simulink model "CCMLin2Mgr"  (R2022b) (continued)
== system CCMLin2Mgr/Lin2Mgr_Runnable_sys == (continued)
  SysServCarCfgSts_SysServDUDCSTLSts_read/1 -> Terminator_18/1
  SysServCarCfgSts_SysServDUDCTLSts_read/1 -> Terminator_19/1
  SysServCarCfgSts_SysServDUDCTRSts_read/1 -> Terminator_20/1
  SysServCarCfgSts_SysServDUFLFootSts_read/1 -> Terminator_21/1
  SysServCarCfgSts_SysServDUFLVentSts_read/1 -> Terminator_22/1
  SysServCarCfgSts_SysServDUFRFootSts_read/1 -> Terminator_23/1
  SysServCarCfgSts_SysServDUFRVentSts_read/1 -> Terminator_24/1
  SysServCarCfgSts_SysServDUIncarMotorSts_read/1 -> Terminator_25/1
  SysServCarCfgSts_SysServDULin1HBMFSts_read/1 -> Terminator_26/1
  SysServCarCfgSts_SysServDULin4HFAFSts_read/1 -> Terminator_27/1
  SysServCarCfgSts_SysServDUSFLFootSts_read/1 -> Terminator_28/1
  SysServCarCfgSts_SysServDUSFLVentSts_read/1 -> Terminator_29/1
  SysServCarCfgSts_SysServDUSFRFootSts_read/1 -> Terminator_30/1
  SysServCarCfgSts_SysServDUSFRVentSts_read/1 -> Terminator_31/1
  SysServCarCfgSts_SysServDUSnsrForFCmptmtTSts_read/1 -> Terminator_32/1
  SysServCarCfgSts_SysServDUSnsrForRair_read/1 -> Terminator_33/1
  SysServCarCfgSts_SysServDUSnsrForRCmptmtTSts_read/1 -> Terminator_34/1
  AACAirClngErrSts_AACAirClngErrSts_read/1 -> Terminator_35/1
  AFUGenErr_AFUGenErr_read/1 -> Terminator_36/1
  AFUPartNo10Cmpl_AFUPartNo10Cmpl_read/1 -> Terminator_37/1
  AFUPartNoCmpl_AFUPartNoCmpl_read/1 -> Terminator_38/1
  AFUSerNo_AFUSerNo_read/1 -> Terminator_39/1
  AirClngErrSts_AirClngErrSts_read/1 -> Terminator_40/1
  AirFragFanErr_AirFragFanErr_read/1 -> Terminator_41/1
  AirFragMot1Err_AirFragMot1Err_read/1 -> Terminator_42/1
  AirFragMot2Err_AirFragMot2Err_read/1 -> Terminator_43/1
  AirFragMot3Err_AirFragMot3Err_read/1 -> Terminator_44/1
  AirFragMot4Err_AirFragMot4Err_read/1 -> Terminator_45/1
  AirFragMot5Err_AirFragMot5Err_read/1 -> Terminator_46/1
  AirFragOvrVoltErr_AirFragOvrVoltErr_read/1 -> Terminator_47/1
  AirFragTempErr_AirFragTempErr_read/1 -> Terminator_48/1
  AirFragUndVoltErr_AirFragUndVoltErr_read/1 -> Terminator_49/1
  AIUPartNo10Cmpl_AIUPartNo10Cmpl_read/1 -> Terminator_50/1
  AIUPartNoCmpl_AIUPartNoCmpl_read/1 -> Terminator_51/1
  AIUSerNo_AIUSerNo_read/1 -> Terminator_52/1
  AQSAirQuality_AQSAirQuality_read/1 -> Terminator_53/1
  AQSCOxstatus_AQSCOxstatus_read/1 -> Terminator_54/1
  AQSNH3status_AQSNH3status_read/1 -> Terminator_55/1
  AQSNoxStatus_AQSNoxStatus_read/1 -> Terminator_56/1
  CarCfgForClimaCtrl_CarCfgForAirClng_read/1 -> Terminator_57/1
  CarCfgForClimaCtrl_CarCfgForAirClnrAdv_read/1 -> Terminator_58/1
  CarCfgForClimaCtrl_CarCfgForAirFrag_read/1 -> Terminator_59/1
  CarCfgForClimaCtrl_CarCfgForAirMtrlSnsr_read/1 -> Terminator_60/1
  CarCfgForClimaCtrl_CarCfgForAirQly_read/1 -> Terminator_61/1
  CarCfgForClimaCtrl_CarCfgForAmntOfSeat_read/1 -> Terminator_62/1
  CarCfgForClimaCtrl_CarCfgForAutDemst_read/1 -> Terminator_63/1
  CarCfgForClimaCtrl_CarCfgForAuxWtrPmp_read/1 -> Terminator_64/1
  CarCfgForClimaCtrl_CarCfgForBRAND_read/1 -> Terminator_65/1
  CarCfgForClimaCtrl_CarCfgForBypVlvInHvac_read/1 -> Terminator_66/1
  CarCfgForClimaCtrl_CarCfgForCabinOxgenSupply_read/1 -> Terminator_67/1
  CarCfgForClimaCtrl_CarCfgForClsVlvInHvac_read/1 -> Terminator_68/1
  CarCfgForClimaCtrl_CarCfgForCmprAcTyp_read/1 -> Terminator_69/1
  CarCfgForClimaCtrl_CarCfgForCmptmtClng_read/1 -> Terminator_70/1
  CarCfgForClimaCtrl_CarCfgForElectricLiftingModule_read/1 -> Terminator_71/1
  CarCfgForClimaCtrl_CarCfgForElecVentAdjmt_read/1 -> Terminator_72/1
  CarCfgForClimaCtrl_CarCfgForExtVentnClimaCtrl_read/1 -> Terminator_73/1
  CarCfgForClimaCtrl_CarCfgForFlcTyp_read/1 -> Terminator_74/1
  CarCfgForClimaCtrl_CarCfgForFoldCSD_read/1 -> Terminator_75/1
  CarCfgForClimaCtrl_CarCfgForFuTyp_read/1 -> Terminator_76/1
  CarCfgForClimaCtrl_CarCfgForGlassTyp_read/1 -> Terminator_77/1
  CarCfgForClimaCtrl_CarCfgForHeadRadar_read/1 -> Terminator_78/1
  CarCfgForClimaCtrl_CarCfgForHeatPmp_read/1 -> Terminator_79/1
  CarCfgForClimaCtrl_CarCfgForHeatrDurgDrvg_read/1 -> Terminator_80/1
  CarCfgForClimaCtrl_CarCfgForHeatrTyp_read/1 -> Terminator_81/1
  CarCfgForClimaCtrl_CarCfgForHvacFilTyp_read/1 -> Terminator_82/1
  CarCfgForClimaCtrl_CarCfgForHvacFlrSnsrForT_read/1 -> Terminator_83/1
  CarCfgForClimaCtrl_CarCfgForHvacHeatrSnsrT_read/1 -> Terminator_84/1
  CarCfgForClimaCtrl_CarCfgForHvacRe_read/1 -> Terminator_85/1
  CarCfgForClimaCtrl_CarCfgForHvacTyp_read/1 -> Terminator_86/1
  CarCfgForClimaCtrl_CarCfgForInteriorViewCamera_read/1 -> Terminator_87/1
  CarCfgForClimaCtrl_CarCfgForLifeDetection_read/1 -> Terminator_88/1
  CarCfgForClimaCtrl_CarCfgForLoVoltElecCfg_read/1 -> Terminator_89/1
  CarCfgForClimaCtrl_CarCfgForNavSysandIntnmap_read/1 -> Terminator_90/1
  CarCfgForClimaCtrl_CarCfgForNrOfDoor_read/1 -> Terminator_91/1
  CarCfgForClimaCtrl_CarCfgForPipeInFuTank_read/1 -> Terminator_92/1
  CarCfgForClimaCtrl_CarCfgForPowerSeats_read/1 -> Terminator_93/1
  CarCfgForClimaCtrl_CarCfgForPreClng_read/1 -> Terminator_94/1
  CarCfgForClimaCtrl_CarCfgForPrkgCDSNotAvl_read/1 -> Terminator_95/1
  CarCfgForClimaCtrl_CarCfgForPrkgClima_read/1 -> Terminator_96/1
  CarCfgForClimaCtrl_CarCfgForPrpsnTyp_read/1 -> Terminator_97/1
  CarCfgForClimaCtrl_CarCfgForPTCInFrontHVAC_read/1 -> Terminator_98/1
  CarCfgForClimaCtrl_CarCfgForRemEngStrtPreCdn_read/1 -> Terminator_99/1
  CarCfgForClimaCtrl_CarCfgForRoofHatch_read/1 -> Terminator_100/1
  CarCfgForClimaCtrl_CarCfgForSeatHeatgCal_read/1 -> Terminator_101/1
  CarCfgForClimaCtrl_CarCfgForSeatHeatgForRowFirst_read/1 -> Terminator_102/1
  CarCfgForClimaCtrl_CarCfgForSeatHeatgForRowSec_read/1 -> Terminator_103/1
  CarCfgForClimaCtrl_CarCfgForSeatVentnForRowFirst_read/1 -> Terminator_104/1
  CarCfgForClimaCtrl_CarCfgForSeatVentnForRowSec_read/1 -> Terminator_105/1
  CarCfgForClimaCtrl_CarCfgForSecRowVentnCenter_read/1 -> Terminator_106/1
  CarCfgForClimaCtrl_CarCfgForSecRowVentnSide_read/1 -> Terminator_107/1
  CarCfgForClimaCtrl_CarCfgForSmallFlowCyc_read/1 -> Terminator_108/1
  CarCfgForClimaCtrl_CarCfgForSmkSnsr_read/1 -> Terminator_109/1
  CarCfgForClimaCtrl_CarCfgForSnsrForCmptmtT_read/1 -> Terminator_110/1
  CarCfgForClimaCtrl_CarCfgForSnsrHumPrsnt_read/1 -> Terminator_111/1
  CarCfgForClimaCtrl_CarCfgForStartDevice_read/1 -> Terminator_112/1
  CarCfgForClimaCtrl_CarCfgForSteerWhlHeatg_read/1 -> Terminator_113/1
  CarCfgForClimaCtrl_CarCfgForSteerWhlPosn_read/1 -> Terminator_114/1
  CarCfgForClimaCtrl_CarCfgForSunSnsr_read/1 -> Terminator_115/1
  CarCfgForClimaCtrl_CarCfgForTelmNodTyp_read/1 -> Terminator_116/1
  CarCfgForClimaCtrl_CarCfgForThermMngt_read/1 -> Terminator_117/1
  CarCfgForClimaCtrl_CarCfgForVehTyp_read/1 -> Terminator_118/1
  CarCfgForClimaCtrl_CarCfgForVentLiftMotorExiSts_read/1 -> Terminator_119/1
  CarCfgForClimaCtrl_CarCfgForVgaMotorExistSts_read/1 -> Terminator_120/1
  CarCfgForClimaCtrl_CarCfgForWadingAssistSystem_read/1 -> Terminator_121/1
  CarCfgForClimaCtrl_CarCfgForWindHeatd_read/1 -> Terminator_122/1
  CarCfgForClimaCtrl_CarCfgWindFollowHumanMov_read/1 -> Terminator_123/1
  CDSPartNo10Cmpl_CDSPartNo10Cmpl_read/1 -> Terminator_124/1
  CDSPartNoCmpl_CDSPartNoCmpl_read/1 -> Terminator_125/1
  CDSSerNo_CDSSerNo_read/1 -> Terminator_126/1
  ClimRlyCmd_ClimRlyCmd_read/1 -> Terminator_127/1
  CmpmtInAirPmAQI_CmpmtInAirPmAQI_read/1 -> Terminator_128/1
  CmpmtInAirPmDnsty_CmpmtInAirPmDnsty_read/1 -> Terminator_129/1
  CmptmtOxygenConcQFNgcc_CmptmtOxygenConcQFNgcc_read/1 -> Terminator_130/1
  CmptmtOxygenConcVluNgcc_CmptmtOxygenConcVluNgcc_read/1 -> Terminator_131/1
  HvacCfgForClimaCtrl_HvacCfgForAQSTypes_read/1 -> Terminator_132/1
  HvacCfgForClimaCtrl_HvacCfgForHvacFrontBlowerType_read/1 -> Terminator_133/1
  HvacCfgForClimaCtrl_HvacCfgForHvacLowSideRETXVESts_read/1 -> Terminator_134/1
  HvacCfgForClimaCtrl_HvacCfgForHvacMiscExistSts_read/1 -> Terminator_135/1
  HvacCfgForClimaCtrl_HvacCfgForHvacMotorExistSts_read/1 -> Terminator_136/1
  HvacCfgForClimaCtrl_HvacCfgForHvacReBlowerType_read/1 -> Terminator_137/1
  HvacCfgForClimaCtrl_HvacCfgForHvacReVentPstn_read/1 -> Terminator_138/1
  HvacCfgForClimaCtrl_HvacCfgForHvacSensorExistSts_read/1 -> Terminator_139/1
  HvacCfgForClimaCtrl_HvacCfgForHvacSnsrRCmptmtESts_read/1 -> Terminator_140/1
  HvacCfgForClimaCtrl_HvacCfgForHvacSnsrReHexESts_read/1 -> Terminator_141/1
  HvacFanReqRe_HvacFanReqRe_read/1 -> Terminator_142/1
  HvacFanSts2HvacFanBattU2_HvacFanSts2HvacFanBattU2_read/1 -> Terminator_143/1
  HvacFanSts2HvacFanExt2_HvacFanSts2HvacFanExt2_read/1 -> Terminator_144/1
  HvacFanSts2HvacFanHw2_HvacFanSts2HvacFanHw2_read/1 -> Terminator_145/1
  HvacFanSts2HvacFanMotU2_HvacFanSts2HvacFanMotU2_read/1 -> Terminator_146/1
  HvacFanSts2HvacFanReSts1_HvacFanSts2HvacFanReSts1_read/1 -> Terminator_147/1
  HvacFanSts2HvacFanReSts2_HvacFanSts2HvacFanReSts2_read/1 -> Terminator_148/1
  HvacFanSts2HvacFanSw2_HvacFanSts2HvacFanSw2_read/1 -> Terminator_149/1
  HvacFanSts2HvacFanT2_HvacFanSts2HvacFanT2_read/1 -> Terminator_150/1
  HvacFanVoltReqRe_HvacFanVoltReqRe_read/1 -> Terminator_151/1
  InPm25FanErr_InPm25FanErr_read/1 -> Terminator_152/1
  InPm25SensrIntErr_InPm25SensrIntErr_read/1 -> Terminator_153/1
  InPm25SnrTempErr_InPm25SnrTempErr_read/1 -> Terminator_154/1
  InPm25SnrVoltgRangErr_InPm25SnrVoltgRangErr_read/1 -> Terminator_155/1
  InPm25SnsrElecErr_InPm25SnsrElecErr_read/1 -> Terminator_156/1
  InPm25SnsrSts_InPm25SnsrSts_read/1 -> Terminator_157/1
  IntCo2CircElecErr_IntCo2CircElecErr_read/1 -> Terminator_158/1
  IntCo2HvErr_IntCo2HvErr_read/1 -> Terminator_159/1
  IntCo2LvErr_IntCo2LvErr_read/1 -> Terminator_160/1
  KeepComActvL2_KeepComActvL2_read/1 -> Terminator_161/1
  OxygenConcSnsrElecErr_OxygenConcSnsrElecErr_read/1 -> Terminator_162/1
  OxygenConcSnsrElecErrNgcc_OxygenConcSnsrElecErrNgcc_read/1 -> Terminator_163/1
  OxygenConcSnsrIntErr_OxygenConcSnsrIntErr_read/1 -> Terminator_164/1
  OxygenConcSnsrIntErrNgcc_OxygenConcSnsrIntErrNgcc_read/1 -> Terminator_165/1
  OxygenConcSnsrOvrVoltRangErr_OxygenConcSnsrOvrVoltRangErr_read/1 -> Terminator_166/1
  OxygenConcSnsrStsNgcc_OxygenConcSnsrStsNgcc_read/1 -> Terminator_167/1
  OxygenConcSnsrTempErr_OxygenConcSnsrTempErr_read/1 -> Terminator_168/1
  OxygenConcSnsrTempErrNgcc_OxygenConcSnsrTempErrNgcc_read/1 -> Terminator_169/1
  OxygenConcSnsrUndVoltRangErr_OxygenConcSnsrUndVoltRangErr_read/1 -> Terminator_170/1
  OxygenConcSnsrUndVoltRangErrNgcc_OxygenConcSnsrUndVoltRangErrNgcc_read/1 -> Terminator_171/1
  PMSIPartNo10Hw_PMSIPartNo10Hw_read/1 -> Terminator_172/1
  PMSIPartNoHw_PMSIPartNoHw_read/1 -> Terminator_173/1
  PwrSplyToSnsrForAirQly_PwrSplyToSnsrForAirQly_read/1 -> Terminator_174/1
  RHFAScheTableReq_Val_read/1 -> Terminator_175/1
  SerNoPMSI_SerNoPMSI_read/1 -> Terminator_176/1
  SysServCarCfgSts_SysServCCPBGMNum_read/1 -> Terminator_177/1
  SysServCarCfgSts_SysServCCPLin2AQSSts_read/1 -> Terminator_178/1
  SysServCarCfgSts_SysServDUDCFLMdeSts_read/1 -> Terminator_179/1
  SysServCarCfgSts_SysServDUReBlowerPwmSignSts_read/1 -> Terminator_180/1
  SysServCarCfgSts_SysServDUReBlowerPwmSts_read/1 -> Terminator_181/1
  SysServCarCfgSts_SysServFrntHEXSnsrPosn_read/1 -> Terminator_182/1
  SysServCarCfgSts_SysServLowSideRETXVSts_read/1 -> Terminator_183/1
  SysServCarCfgSts_SysServReHEXSnsrPosn_read/1 -> Terminator_184/1
  SysServCarCfgSts_SysServRHFASts_read/1 -> Terminator_185/1
  SysServCarCfgSts_SysServSnsrForFCmptmtTType_read/1 -> Terminator_186/1
  BswLifeCycle_LifeCycle_read/1 -> Terminator_187/1
  Ground_1/1 -> LinIfServ_GetScheduleInfo/1
  LinIfServ_GetScheduleInfo/1 -> Terminator_188/1
  LinIfServ_GetScheduleInfo/2 -> Terminator_189/1
  Ground_2/1 -> LinSMServ_ScheduleRequest/1
  Ground_3/1 -> LinSMServ_ScheduleRequest/2
  LinSMServ_ScheduleRequest/1 -> Terminator_190/1
  LinService_GetLin2WakeupStatus/1 -> Terminator_191/1
  LinService_GetLin2WakeupStatus/2 -> Terminator_192/1
  Ground_4/1 -> LinService_SendLin3dFrame/1
  Ground_5/1 -> LinService_SendLin3dFrame/2
  LinService_SendLin3dFrame/1 -> Terminator_193/1
  Ground_6/1 -> LinService_SetLin3cFrame/1
  Ground_7/1 -> LinService_SetLin3cFrame/2
  LinService_SetLin3cFrame/1 -> Terminator_194/1
  Ground_8/1 -> LinService_SetLinTable/1
  Ground_9/1 -> LinService_SetLinTable/2
  LinService_SetLinTable/1 -> Terminator_195/1
  MirrorLin2ErrServ_GetAQSCcmLin2Fr01NoResp/1 -> Terminator_196/1
  MirrorLin2ErrServ_GetAQSRxErrSta/1 -> Terminator_197/1
  MirrorLin2ErrServ_GetAfuCcmLin2Fr01NoResp/1 -> Terminator_198/1
  MirrorLin2ErrServ_GetAfuCcmLin2Fr01NoResp/2 -> Terminator_199/1
  MirrorLin2ErrServ_GetAfuRxErrSta/1 -> Terminator_200/1
  MirrorLin2ErrServ_GetAfuRxErrSta/2 -> Terminator_201/1
  MirrorLin2ErrServ_GetAiuCcmLin2Fr01NoResp/1 -> Terminator_202/1
  MirrorLin2ErrServ_GetAiuCcmLin2Fr01NoResp/2 -> Terminator_203/1
  MirrorLin2ErrServ_GetAiuRxErrSta/1 -> Terminator_204/1
  MirrorLin2ErrServ_GetAiuRxErrSta/2 -> Terminator_205/1
  MirrorLin2ErrServ_GetCdsCcmLin2Fr01NoResp/1 -> Terminator_206/1
  MirrorLin2ErrServ_GetCdsCcmLin2Fr01NoResp/2 -> Terminator_207/1
  MirrorLin2ErrServ_GetCdsRxErrSta/1 -> Terminator_208/1
  MirrorLin2ErrServ_GetCdsRxErrSta/2 -> Terminator_209/1
  MirrorLin2ErrServ_GetLinBusTxErrSta/1 -> Terminator_210/1
  MirrorLin2ErrServ_GetLinBusTxErrSta/2 -> Terminator_211/1
  MirrorLin2ErrServ_GetPmsiCcmLin2Fr01NoResp/1 -> Terminator_212/1
  MirrorLin2ErrServ_GetPmsiCcmLin2Fr01NoResp/2 -> Terminator_213/1
  MirrorLin2ErrServ_GetPmsiRxErrSta/1 -> Terminator_214/1
  MirrorLin2ErrServ_GetPmsiRxErrSta/2 -> Terminator_215/1
  MirrorLin2ErrServ_GetRhfaCcmLin2Fr01NoResp/1 -> Terminator_216/1
  MirrorLin2ErrServ_GetRhfaCcmLin2Fr01NoResp/2 -> Terminator_217/1
  MirrorLin2ErrServ_GetRhfaRxErrSta/1 -> Terminator_218/1
  MirrorLin2ErrServ_GetRhfaRxErrSta/2 -> Terminator_219/1
  MirrorLin2ErrServ_GetVbmrCcmLin2Fr01NoResp/1 -> Terminator_220/1
  MirrorLin2ErrServ_GetVbmrCcmLin2Fr01NoResp/2 -> Terminator_221/1
  MirrorLin2ErrServ_GetVbmrRxErrSta/1 -> Terminator_222/1
  MirrorLin2ErrServ_GetVbmrRxErrSta/2 -> Terminator_223/1
  Ground_10/1(Out1) -> AirQlyLin_AirQlyLin_write/1
  Ground_11/1 -> HvacFanSet2HvacFanRxFrq2_HvacFanSet2HvacFanRxFrq2_write/1
  Ground_12/1 -> Lin2ActvReady_Lin2ActvReady_write/1
  Ground_13/1 -> Lin2MgrDtcServ2_AACGenElectrFail_write/1
  Ground_14/1 -> Lin2MgrDtcServ2_IOSMGenElectrFail_write/1
  Ground_15/1 -> Lin2MgrDtcServ2_IOSMInterFail_write/1
  Ground_16/1 -> ReVbmrSts_ReVbmrSts_write/1
  Ground_17/1 -> AirFragErrSts_AirFragErrSts_write/1
  Ground_18/1 -> AirPmQf_AirPmQf_write/1
  Ground_19/1 -> HvacFanSet2HvacFanIMaxMax2_HvacFanSet2HvacFanIMaxMax2_write/1
  Ground_20/1 -> HvacFanSet2HvacFanIMaxMin2_HvacFanSet2HvacFanIMaxMin2_write/1
  Ground_21/1 -> HvacFanSet2HvacFanKneeU2_HvacFanSet2HvacFanKneeU2_write/1
  Ground_22/1 -> HvacFanSet2HvacFanRamp2_HvacFanSet2HvacFanRamp2_write/1
  Ground_23/1 -> HvacFanSet2HvacFanSeln2_HvacFanSet2HvacFanSeln2_write/1
  Ground_24/1 -> HvacFanSet2HvacFanSftyRunThd2_HvacFanSet2HvacFanSftyRunThd2_write/1
  Ground_25/1 -> HvacFanSet2HvacFanSpd2_HvacFanSet2HvacFanSpd2_write/1
  Ground_26/1 -> HvacFanSet2HvacSftyRunSpd2_HvacFanSet2HvacSftyRunSpd2_write/1
  Ground_27/1 -> IntPm25LvlFrmClima_IntPm25LvlFrmClima_write/1
  Ground_28/1 -> IntPm25StsFrmClima_IntPm25StsFrmClima_write/1
  Ground_29/1 -> IntPm25VluFrmClima_IntPm25VluFrmClima_write/1
  Ground_30/1 -> LIN2ScheTableReq_Val_write/1

== system CCMLin2Mgr/Lin2Mgr_SerNoPMSI_sys ==
  SerNoPMSI_SerNoPMSI_read : Inport
  function : TriggerPort  TriggerType=function-call  VariantControl=(inherit)
  Terminator_1 : Terminator
  wires:
  SerNoPMSI_SerNoPMSI_read/1 -> Terminator_1/1

== system CCMLin2Mgr/Lin2ParaService ==
  Lin2ParaService_FreezeVbmrReqPercent_sys : Subsystem
  Lin2ParaService_ReadVbmrPercent_sys : Subsystem
  Lin2ParaService_VbmrReqPercentRetCtrltoECU_sys : Subsystem
  Lin2ParaService_VbmrReqPercentShortTermAdj_sys : Subsystem

== system CCMLin2Mgr/Lin2MgrDtcServ/Lin2MgrDtcServ_AFUGenElectrFail_sys ==
  function : TriggerPort  TriggerType=function-call  IsSimulinkFunction=on  FunctionName=Lin2MgrDtcServ_AFUGenElectrFail  VariantControl=(inherit)
  Ground_1 : Ground
  Ground_2 : Ground
  Status : ArgOut  OutDataTypeStr=UInt8  ArgumentName=Status
  ERR : ArgOut  Port=2  OutDataTypeStr=Std_ReturnType  ArgumentName=ERR
  wires:
  Ground_1/1 -> Status/1
  Ground_2/1 -> ERR/1

== system CCMLin2Mgr/Lin2MgrDtcServ/Lin2MgrDtcServ_AFUInterFail_sys ==
  function : TriggerPort  TriggerType=function-call  IsSimulinkFunction=on  FunctionName=Lin2MgrDtcServ_AFUInterFail  VariantControl=(inherit)
  Ground_1 : Ground
  Ground_2 : Ground
  Ststus : ArgOut  OutDataTypeStr=UInt8  ArgumentName=Ststus
  ERR : ArgOut  Port=2  OutDataTypeStr=Std_ReturnType  ArgumentName=ERR
  wires:
  Ground_1/1 -> Ststus/1
  Ground_2/1 -> ERR/1

== system CCMLin2Mgr/Lin2MgrDtcServ/Lin2MgrDtcServ_AIUGenElectrFail_sys ==
  function : TriggerPort  TriggerType=function-call  IsSimulinkFunction=on  FunctionName=Lin2MgrDtcServ_AIUGenElectrFail  VariantControl=(inherit)
  Ground_1 : Ground
  Ground_2 : Ground
  Status : ArgOut  OutDataTypeStr=UInt8  ArgumentName=Status
  ERR : ArgOut  Port=2  OutDataTypeStr=Std_ReturnType  ArgumentName=ERR
  wires:
  Ground_1/1 -> Status/1
  Ground_2/1 -> ERR/1

== system CCMLin2Mgr/Lin2MgrDtcServ/Lin2MgrDtcServ_AQSInterFail_sys ==
  function : TriggerPort  TriggerType=function-call  IsSimulinkFunction=on  FunctionName=Lin2MgrDtcServ_AQSInterFail  VariantControl=(inherit)
  Ground_1 : Ground
  Ground_2 : Ground
  Status : ArgOut  OutDataTypeStr=UInt8  ArgumentName=Status
  ERR : ArgOut  Port=2  OutDataTypeStr=Std_ReturnType  ArgumentName=ERR
  wires:
  Ground_1/1 -> Status/1
  Ground_2/1 -> ERR/1

== system CCMLin2Mgr/Lin2MgrDtcServ/Lin2MgrDtcServ_CDSGenElectrFail_sys ==
  function : TriggerPort  TriggerType=function-call  IsSimulinkFunction=on  FunctionName=Lin2MgrDtcServ_CDSGenElectrFail  VariantControl=(inherit)
  Ground_1 : Ground
  Ground_2 : Ground
  Status : ArgOut  OutDataTypeStr=UInt8  ArgumentName=Status
  ERR : ArgOut  Port=2  OutDataTypeStr=Std_ReturnType  ArgumentName=ERR
  wires:
  Ground_1/1 -> Status/1
  Ground_2/1 -> ERR/1

== system CCMLin2Mgr/Lin2MgrDtcServ/Lin2MgrDtcServ_PMSIGenElectrFail_sys ==
  function : TriggerPort  TriggerType=function-call  IsSimulinkFunction=on  FunctionName=Lin2MgrDtcServ_PMSIGenElectrFail  VariantControl=(inherit)
  Ground_1 : Ground
  Ground_2 : Ground
  Status : ArgOut  OutDataTypeStr=UInt8  ArgumentName=Status
  ERR : ArgOut  Port=2  OutDataTypeStr=Std_ReturnType  ArgumentName=ERR
  wires:
  Ground_1/1 -> Status/1
  Ground_2/1 -> ERR/1

== system CCMLin2Mgr/Lin2MgrDtcServ/Lin2MgrDtcServ_PMSIInterFail_sys ==
  function : TriggerPort  TriggerType=function-call  IsSimulinkFunction=on  FunctionName=Lin2MgrDtcServ_PMSIInterFail  VariantControl=(inherit)
  Ground_1 : Ground
  Ground_2 : Ground
  Status : ArgOut  OutDataTypeStr=UInt8  ArgumentName=Status
  ERR : ArgOut  Port=2  OutDataTypeStr=Std_ReturnType  ArgumentName=ERR
  wires:
  Ground_1/1 -> Status/1
  Ground_2/1 -> ERR/1

== system CCMLin2Mgr/Lin2MgrDtcServ/Lin2MgrDtcServ_VBMRGenElectrFail_sys ==
  function : TriggerPort  TriggerType=function-call  IsSimulinkFunction=on  FunctionName=Lin2MgrDtcServ_VBMRGenElectrFail  VariantControl=(inherit)
  Ground_1 : Ground
  Ground_2 : Ground
  Status : ArgOut  OutDataTypeStr=UInt8  ArgumentName=Status
  ERR : ArgOut  Port=2  OutDataTypeStr=Std_ReturnType  ArgumentName=ERR
  wires:
  Ground_1/1 -> Status/1
  Ground_2/1 -> ERR/1

== system CCMLin2Mgr/Lin2MgrDtcServ/Lin2MgrDtcServ_VBMRInterFail_sys ==
  function : TriggerPort  TriggerType=function-call  IsSimulinkFunction=on  FunctionName=Lin2MgrDtcServ_VBMRInterFail  VariantControl=(inherit)
  Ground_1 : Ground
  Ground_2 : Ground
  Status : ArgOut  OutDataTypeStr=UInt8  ArgumentName=Status
  ERR : ArgOut  Port=2  OutDataTypeStr=Std_ReturnType  ArgumentName=ERR
  wires:
  Ground_1/1 -> Status/1
  Ground_2/1 -> ERR/1

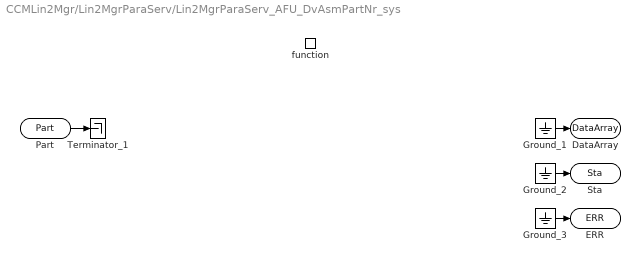
== system CCMLin2Mgr/Lin2MgrParaServ/Lin2MgrParaServ_AFU_DvAsmPartNr_sys ==
  function : TriggerPort  TriggerType=function-call  IsSimulinkFunction=on  FunctionName=Lin2MgrParaServ_AFU_DvAsmPartNr  VariantControl=(inherit)
  Ground_1 : Ground
  Ground_2 : Ground
  Ground_3 : Ground
  Terminator_1 : Terminator
  Part : ArgIn  OutDataTypeStr=UInt8  ArgumentName=Part
  DataArray : ArgOut  OutDataTypeStr=UInt8  PortDimensions=5  ArgumentName=DataArray
  Sta : ArgOut  Port=2  OutDataTypeStr=UInt8  ArgumentName=Sta
  ERR : ArgOut  Port=3  OutDataTypeStr=Std_ReturnType  ArgumentName=ERR
  wires:
  Part/1 -> Terminator_1/1
  Ground_1/1 -> DataArray/1
  Ground_2/1 -> Sta/1
  Ground_3/1 -> ERR/1

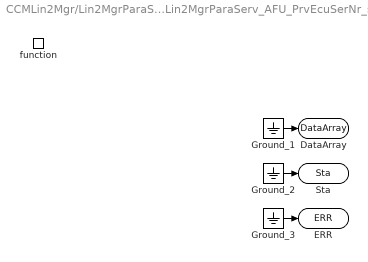
== system CCMLin2Mgr/Lin2MgrParaServ/Lin2MgrParaServ_AFU_PrvEcuSerNr_sys ==
  function : TriggerPort  TriggerType=function-call  IsSimulinkFunction=on  FunctionName=Lin2MgrParaServ_AFU_PrvEcuSerNr  VariantControl=(inherit)
  Ground_1 : Ground
  Ground_2 : Ground
  Ground_3 : Ground
  DataArray : ArgOut  OutDataTypeStr=UInt8  PortDimensions=5  ArgumentName=DataArray
  Sta : ArgOut  Port=2  OutDataTypeStr=UInt8  ArgumentName=Sta
  ERR : ArgOut  Port=3  OutDataTypeStr=Std_ReturnType  ArgumentName=ERR
  wires:
  Ground_1/1 -> DataArray/1
  Ground_2/1 -> Sta/1
  Ground_3/1 -> ERR/1

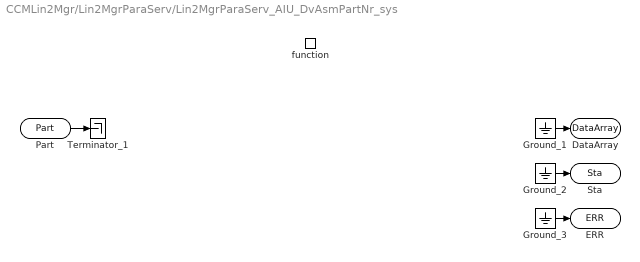
== system CCMLin2Mgr/Lin2MgrParaServ/Lin2MgrParaServ_AIU_DvAsmPartNr_sys ==
  function : TriggerPort  TriggerType=function-call  IsSimulinkFunction=on  FunctionName=Lin2MgrParaServ_AIU_DvAsmPartNr  VariantControl=(inherit)
  Ground_1 : Ground
  Ground_2 : Ground
  Ground_3 : Ground
  Terminator_1 : Terminator
  Part : ArgIn  OutDataTypeStr=UInt8  ArgumentName=Part
  DataArray : ArgOut  OutDataTypeStr=UInt8  PortDimensions=5  ArgumentName=DataArray
  Sta : ArgOut  Port=2  OutDataTypeStr=UInt8  ArgumentName=Sta
  ERR : ArgOut  Port=3  OutDataTypeStr=Std_ReturnType  ArgumentName=ERR
  wires:
  Part/1 -> Terminator_1/1
  Ground_1/1 -> DataArray/1
  Ground_2/1 -> Sta/1
  Ground_3/1 -> ERR/1

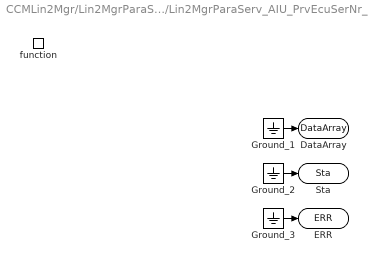
== system CCMLin2Mgr/Lin2MgrParaServ/Lin2MgrParaServ_AIU_PrvEcuSerNr_sys ==
  function : TriggerPort  TriggerType=function-call  IsSimulinkFunction=on  FunctionName=Lin2MgrParaServ_AIU_PrvEcuSerNr  VariantControl=(inherit)
  Ground_1 : Ground
  Ground_2 : Ground
  Ground_3 : Ground
  DataArray : ArgOut  OutDataTypeStr=UInt8  PortDimensions=5  ArgumentName=DataArray
  Sta : ArgOut  Port=2  OutDataTypeStr=UInt8  ArgumentName=Sta
  ERR : ArgOut  Port=3  OutDataTypeStr=Std_ReturnType  ArgumentName=ERR
  wires:
  Ground_1/1 -> DataArray/1
  Ground_2/1 -> Sta/1
  Ground_3/1 -> ERR/1

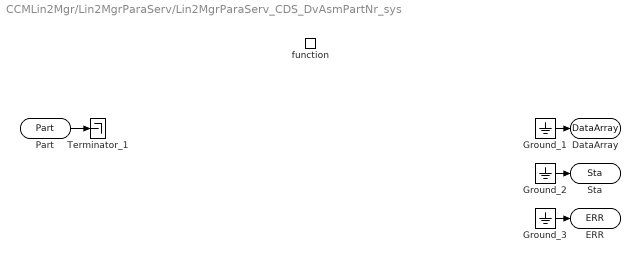
== system CCMLin2Mgr/Lin2MgrParaServ/Lin2MgrParaServ_CDS_DvAsmPartNr_sys ==
  function : TriggerPort  TriggerType=function-call  IsSimulinkFunction=on  FunctionName=Lin2MgrParaServ_CDS_DvAsmPartNr  VariantControl=(inherit)
  Ground_1 : Ground
  Ground_2 : Ground
  Ground_3 : Ground
  Terminator_1 : Terminator
  Part : ArgIn  OutDataTypeStr=UInt8  ArgumentName=Part
  DataArray : ArgOut  OutDataTypeStr=UInt8  PortDimensions=5  ArgumentName=DataArray
  Sta : ArgOut  Port=2  OutDataTypeStr=UInt8  ArgumentName=Sta
  ERR : ArgOut  Port=3  OutDataTypeStr=Std_ReturnType  ArgumentName=ERR
  wires:
  Part/1 -> Terminator_1/1
  Ground_1/1 -> DataArray/1
  Ground_2/1 -> Sta/1
  Ground_3/1 -> ERR/1

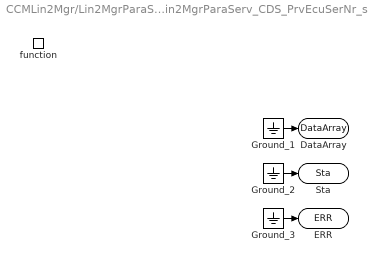
== system CCMLin2Mgr/Lin2MgrParaServ/Lin2MgrParaServ_CDS_PrvEcuSerNr_sys ==
  function : TriggerPort  TriggerType=function-call  IsSimulinkFunction=on  FunctionName=Lin2MgrParaServ_CDS_PrvEcuSerNr  VariantControl=(inherit)
  Ground_1 : Ground
  Ground_2 : Ground
  Ground_3 : Ground
  DataArray : ArgOut  OutDataTypeStr=UInt8  PortDimensions=5  ArgumentName=DataArray
  Sta : ArgOut  Port=2  OutDataTypeStr=UInt8  ArgumentName=Sta
  ERR : ArgOut  Port=3  OutDataTypeStr=Std_ReturnType  ArgumentName=ERR
  wires:
  Ground_1/1 -> DataArray/1
  Ground_2/1 -> Sta/1
  Ground_3/1 -> ERR/1

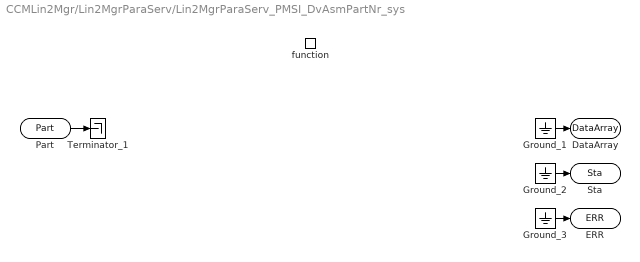
== system CCMLin2Mgr/Lin2MgrParaServ/Lin2MgrParaServ_PMSI_DvAsmPartNr_sys ==
  function : TriggerPort  TriggerType=function-call  IsSimulinkFunction=on  FunctionName=Lin2MgrParaServ_PMSI_DvAsmPartNr  VariantControl=(inherit)
  Ground_1 : Ground
  Ground_2 : Ground
  Ground_3 : Ground
  Terminator_1 : Terminator
  Part : ArgIn  OutDataTypeStr=UInt8  ArgumentName=Part
  DataArray : ArgOut  OutDataTypeStr=UInt8  PortDimensions=5  ArgumentName=DataArray
  Sta : ArgOut  Port=2  OutDataTypeStr=UInt8  ArgumentName=Sta
  ERR : ArgOut  Port=3  OutDataTypeStr=Std_ReturnType  ArgumentName=ERR
  wires:
  Part/1 -> Terminator_1/1
  Ground_1/1 -> DataArray/1
  Ground_2/1 -> Sta/1
  Ground_3/1 -> ERR/1

== system CCMLin2Mgr/Lin2MgrParaServ/Lin2MgrParaServ_PMSI_PrvEcuSerNr_sys ==
  function : TriggerPort  TriggerType=function-call  IsSimulinkFunction=on  FunctionName=Lin2MgrParaServ_PMSI_PrvEcuSerNr  VariantControl=(inherit)
  Ground_1 : Ground
  Ground_2 : Ground
  Ground_3 : Ground
  DataArray : ArgOut  OutDataTypeStr=UInt8  PortDimensions=5  ArgumentName=DataArray
  Sta : ArgOut  Port=2  OutDataTypeStr=UInt8  ArgumentName=Sta
  ERR : ArgOut  Port=3  OutDataTypeStr=Std_ReturnType  ArgumentName=ERR
  wires:
  Ground_1/1 -> DataArray/1
  Ground_2/1 -> Sta/1
  Ground_3/1 -> ERR/1

== system CCMLin2Mgr/Lin2Mgr_Runnable_sys/Ground_10 ==
  Ground_10 : Ground
  Out1 : Outport  OutDataTypeStr=Bus: AirQlyLinDutyWithQly  PortDimensions=1
  wires:
  Ground_10/1 -> Out1/1

== system CCMLin2Mgr/Lin2ParaService/Lin2ParaService_FreezeVbmrReqPercent_sys ==
  function : TriggerPort  TriggerType=function-call  IsSimulinkFunction=on  FunctionName=Lin2ParaService_FreezeVbmrReqPercent  VariantControl=(inherit)
  Ground_1 : Ground
  ERR : ArgOut  OutDataTypeStr=Std_ReturnType  ArgumentName=ERR
  wires:
  Ground_1/1 -> ERR/1
